simulink model "TosAeb"  (R2021a)
settings: Solver=FixedStepDiscrete  StartTime=0.0  StopTime=inf  FixedStep=0.01  RelTol=1e-3
undefined symbols (not defined in the file or in its folder): ACCModSts, AccSts1, AEB_ObjectMovingOrientation, AEB_ObjectMovingState, CN_BTCActive, CN_CurrentGearR, CN_CurrentGearR_DHT, CN_PTCActive, CN_VDCActive, CN_VSC_EspOff, CN_VSC_FCW_AEB_Error, CN_VSC_FCW_AEB_NoDispay, CN_VSC_FCW_AEB_PerfmDgdtn, CN_VSC_FCW_AEB_TempUnAvlbl, CN_VSC_VehStandstill, CNaccelAvoidMax, CNaccelAvoidMin, CNbrakePedalPress, CNcomfortStrLeftAccelMax, CNcomfortStrLeftAccelMin, CNcomfortStrRightAccelMax, CNcomfortStrRightAccelMin, CNDrvDoorOpen, CNDrvModeEco, CNDrvModeNorm, CNDrvModeOther, CNDrvModeSport, CNDrvSBRreminder, CNNull, CNobjInvalidID, CNPassDoorOpen, CNPassSBRreminder, CNPEBSAccelNegInf, CNPEBSAccelNull, CNPEBSTimeInf, CNPEBSTimeNull, CNSTOPMAX, CNSTOPNULL, CNsubjWidth, CNSwtOFF, CNSwtON, CNTTCMAX, CNTTCNULL, CNVehConfigAEBCycNone, CNVehConfigAEBNone, CNVehConfigAEBPedNone, CNVehConfigFCWNone, CNVehConfigJANone, CollisionSeverity, FALSE, FCWSnvty, JACycObjCalcInfoDefaultParams, JAPedObjCalcInfoDefaultParams, JAVehObjCalcInfoDefaultParams, latCycObjCalcInfoDefaultParams, latPedObjCalcInfoDefaultParams, longObjCalcInfoDefaultParams, ModSts, movingStatus, ObjectType ... (+1112)
libraries: simulink x3031, AEBSFunctionLib x50, Ta_Library x33, AEBSStateMachineLib x23, Mylibrary x12, simulink_extras x7, ObjSelect_lib x3, dspstat3 x2
(part 21 of 102)

== system TosAeb/AEB_Objsel/TA_Processor/PreProcessor/VehiclePreProcessor/CalculateCurvature/StaticBicycleModelC0/CalculateCurvatureCoG ==
  note: V^2/R  = w^2 R
      | M w^2 R/Cr = F/ Cr =
  note: We use a bicycle model with the following relations:
      |
      | Lf = Distance from CoG to front axle
      | Lr = Distance from CoG to rear axle
      | B = WheelBase = Lf + Lr
      | R = Curvature radius for CoG
      | M = Vehicle mass
      | V = Vehicle Speed
      | Cf = Cornering stiffness front
      | Cr = Cornering stiffness rear
      |
      | WheelAngle = RearSlipAngle - FrontSlipAngle + B/R
      | RearSlipAngle = - M Lf V^2 / ( B Cr R)
      | FrontSlipAngle = - M Lr V^2 / ( B Cf R )
      |
      | We solve for the curvature radius R, and use that Curvature = 1/R. Then
      | Curvature = WheelAngle B /(B^2 + M V^2 (Lr/Cf - Lf/Cr) )
  WheelAngle : Inport
  DistCoGToRearAxle : Inport  Port=2
  DistCoGToFrontAxle : Inport  Port=3
  MassSpeedSquaredDivWheelBase : Inport  Port=4
  Constant : Constant  Value=P_TA_PrmForVehWhlBase
  Constant1 : Constant  Value=single(1)  OutDataTypeStr=single
  Constant2 : Constant  Value=P_TA_PrmForVehBicycleMdlCornrgStfnFrnt
  Constant3 : Constant  Value=P_TA_PrmForVehBicycleMdlCornrgStfnRe
  Divide2 : Product  Tag=struct('comment',struct('text','','placement',1),'logdata',struct('maxnumvals',inf,'plotchannels',[],'loggingmode',3,'channels',1),'output',struct('variable','','description','','name','$S_$B','width',1,'address','','max',NaN,'min',NaN,'class','default','type','Float32','scaling','','lsb',1,'offset',0,'arb',0,'freeze',1,'unit','','autoscalingmode',1,'lowerheadroom',1,'upperheadroom',1,'headroomunit','bit','keepdatatype',1,'checkmin',0,'checkmax',0,'castoutput',0,'show',0),'inputs','**/','exceptionhandling',1,'scalingvalid',0,'date',734662.46776173613)  Inputs=**
  RadiusCoGJudge : Subsystem
  SafeDivider : Mylibrary/SafeDivider  SystemSampleTime=-1  VariantActivationTime=update diagram  Latency=0  AutoFrameSizeCalculation=off
  SafeDivider1 : Mylibrary/SafeDivider  SystemSampleTime=-1  VariantActivationTime=update diagram  Latency=0  AutoFrameSizeCalculation=off
  SafeDivider2 : Mylibrary/SafeDivider  SystemSampleTime=-1  VariantActivationTime=update diagram  Latency=0  AutoFrameSizeCalculation=off
  Sum1 : Sum  Tag=struct('comment',struct('text','','placement',1),'logdata',struct('maxnumvals',inf,'plotchannels',[],'loggingmode',3,'channels',1),'output',struct('variable','','description','','name','$S_$B','width',1,'address','','max',NaN,'min',NaN,'class','default','type','Float32','scaling','','lsb',1,'offset',0,'arb',0,'freeze',1,'unit','','autoscalingmode',1,'lowerheadroom',1,'upperheadroom',1,'headroomunit','bit','keepdatatype',1,'checkmin',0,'checkmax',0,'castoutput',0,'show',0),'inputs','++','scalingvalid',0,'date',730486)  InputSameDT=on  SaturateOnIntegerOverflow=on  OutDataTypeStr=Inherit: Same as first input
      settings: AttributesFormatString=InputSameDT=%<InputSameDT>
  Sum2 : Sum  Tag=struct('comment',struct('text','','placement',1),'logdata',struct('maxnumvals',inf,'plotchannels',[],'loggingmode',3,'channels',1),'output',struct('variable','','description','','name','$S_$B','width',1,'address','','max',NaN,'min',NaN,'class','default','type','Float32','scaling','','lsb',1,'offset',0,'arb',0,'freeze',1,'unit','','autoscalingmode',1,'lowerheadroom',1,'upperheadroom',1,'headroomunit','bit','keepdatatype',1,'checkmin',0,'checkmax',0,'castoutput',0,'show',0),'inputs','+-','scalingvalid',0,'date',734662.46684667829)  Inputs=+-  InputSameDT=on  SaturateOnIntegerOverflow=on  OutDataTypeStr=Inherit: Same as first input
      settings: AttributesFormatString=InputSameDT=%<InputSameDT>
  RadiusCoG : Outport
  CurvatureCoG : Outport  Port=2
  wires:
  SafeDivider1/1 -> Sum2/2
  DistCoGToFrontAxle/1 -> SafeDivider1/1
  WheelAngle/1 -> SafeDivider2/1
  SafeDivider/1 -> Sum2/1
  DistCoGToRearAxle/1 -> SafeDivider/1
  SafeDivider2/1 -> RadiusCoGJudge/2(CurvatureCoG), CurvatureCoG/1  "CurvatureCoG"
  Sum2/1 -> Divide2/1
  MassSpeedSquaredDivWheelBase/1 -> Divide2/2
  Divide2/1 -> Sum1/2
  Sum1/1 -> SafeDivider2/2
  Constant/1 -> Sum1/1
  Constant2/1 -> SafeDivider/2
  Constant3/1 -> SafeDivider1/2
  Constant1/1 -> RadiusCoGJudge/1(Con)
  RadiusCoGJudge/1(RadiusCoG) -> RadiusCoG/1

== system TosAeb/AEB_Objsel/TA_Processor/PreProcessor/VehiclePreProcessor/CalculateCurvature/StaticBicycleModelC0/CalculateCurvatureRear ==
  RadiusCOG : Inport
  DistCoGToRearAxle : Inport  Port=2
  SlipAngle : Inport  Port=3
  Constant : Constant  Value=single(1)  OutDataTypeStr=single
  Cos : Trigonometry  Operator=cos
  Multipl{,1..4} (5 alike) : Product  Tag=struct('comment',struct('text','','placement',1),'logdata',struct('maxnumvals',inf,'plotchannels',[],'loggingmode',3,'channels',1),'output',struct('variable','','description','','name','$S_$B','width',1,'address','','max',NaN,'min',NaN,'class','LOCAL','type','Float32','scaling','','lsb',1,'offset',0,'arb',0,'freeze',1,'unit','','autoscalingmode',1,'lowerheadroom',1,'upperheadroom',1,'headroomunit','bit','keepdatatype',1,'checkmin',0,'checkmax',0,'castoutput',0,'show',0),'inputs','****','exceptionhandling',1,'scalingvalid',0,'date',734725.68365415512)  InputSameDT=on  RndMeth=Zero  SaturateOnIntegerOverflow=on  OutDataTypeStr=Inherit: Same as first input
  SafeDivider : Mylibrary/SafeDivider  SystemSampleTime=-1  VariantActivationTime=update diagram  Latency=0  AutoFrameSizeCalculation=off
  Sign : Signum
  Sin : Trigonometry
  Sqrt : Sqrt  SaturateOnIntegerOverflow=on
  Sum1 : Sum  Tag=struct('comment',struct('text','','placement',1),'logdata',struct('maxnumvals',inf,'plotchannels',[],'loggingmode',3,'channels',1),'output',struct('variable','','description','','name','$S_$B','width',1,'address','','max',NaN,'min',NaN,'class','default','type','Float32','scaling','','lsb',1,'offset',0,'arb',0,'freeze',1,'unit','','autoscalingmode',1,'lowerheadroom',1,'upperheadroom',1,'headroomunit','bit','keepdatatype',1,'checkmin',0,'checkmax',0,'castoutput',0,'show',0),'inputs','+-','scalingvalid',0,'date',734662.46684667829)  Inputs=+-  InputSameDT=on  SaturateOnIntegerOverflow=on  OutDataTypeStr=Inherit: Same as first input
      settings: AttributesFormatString=InputSameDT=%<InputSameDT>
  Sum3 : Sum  Tag=struct('comment',struct('text','','placement',1),'logdata',struct('maxnumvals',inf,'plotchannels',[],'loggingmode',3,'channels',1),'output',struct('variable','','description','','name','$S_$B','width',1,'address','','max',NaN,'min',NaN,'class','default','type','Float32','scaling','','lsb',1,'offset',0,'arb',0,'freeze',1,'unit','','autoscalingmode',1,'lowerheadroom',1,'upperheadroom',1,'headroomunit','bit','keepdatatype',1,'checkmin',0,'checkmax',0,'castoutput',0,'show',0),'inputs','+-','scalingvalid',0,'date',734662.46684667829)  InputSameDT=on  SaturateOnIntegerOverflow=on  OutDataTypeStr=Inherit: Same as first input
      settings: AttributesFormatString=InputSameDT=%<InputSameDT>
  CurvatureRear : Outport
  wires:
  SlipAngle/1 -> Sin/1, Cos/1
  Sin/1 -> Multipl2/1
  Cos/1 -> Multipl3/1
  RadiusCOG/1 -> Multipl3/2, Sign/1, [RadiusCoG]
  Multipl3/1 -> Multipl/1, Multipl/2  "RadiusCentOfRot"
  DistCoGToRearAxle/1 -> Sum1/1
  Multipl2/1 -> Sum1/2
  Sum1/1 -> Multipl1/1, Multipl1/2
  Multipl1/1 -> Sum3/1
  Multipl/1 -> Sum3/2
  Sum3/1 -> Sqrt/1
  SafeDivider/1 -> CurvatureRear/1  "CurvatureRear"
  Sign/1 -> Multipl4/2
  Sqrt/1 -> Multipl4/1
  [RadiusCoG] -> Multipl2/2
  Multipl4/1 -> SafeDivider/2  "RadiusRear"
  Constant/1 -> SafeDivider/1

== system TosAeb/AEB_Objsel/TA_Processor/PreProcessor/VehiclePreProcessor/CalculateCurvature/StaticBicycleModelC0/CalculateDistanceToAxles ==
  note: 计算质心至后轴中心的距离
  Constant : Constant  Value=P_TA_PrmForVehWhlBase
  Constant1 : Constant  Value=P_TA_PrmForVehBicycleMdlAxleDistFrnt
  Rename : Subsystem  mask(Rename){}
      mask help: Block intended for fooling the model advisor, which want the signal names to match the port names and which only allows signal renaming in combination with transformative operations. The block has no effect on the signal and is intended to replace, e.g., gain blocks with one as its multiplication factor.
      mask Help: Block intended for fooling the model advisor, which want the signal names to match the port names and which only allows signal renaming in combination with transformative operations. The block has no effect on the signal and is intended to replace, e.g., gain blocks with one as its multiplication factor.
      mask Display:
      | % Author: Jakob Andersson (jande573)
      | 
      | % Display the block title
      | disp('Rename');
  Sum2 : Sum  Tag=struct('comment',struct('text','','placement',1),'logdata',struct('maxnumvals',inf,'plotchannels',[],'loggingmode',3,'channels',1),'output',struct('variable','','description','','name','$S_$B','width',1,'address','','max',NaN,'min',NaN,'class','default','type','Float32','scaling','','lsb',1,'offset',0,'arb',0,'freeze',1,'unit','','autoscalingmode',1,'lowerheadroom',1,'upperheadroom',1,'headroomunit','bit','keepdatatype',1,'checkmin',0,'checkmax',0,'castoutput',0,'show',0),'inputs','+-','scalingvalid',0,'date',734662.46684667829)  Inputs=+-  InputSameDT=on  SaturateOnIntegerOverflow=on  OutDataTypeStr=Inherit: Same as first input
      settings: AttributesFormatString=InputSameDT=%<InputSameDT>
  DistCoGToRearAxle : Outport
  DistCoGToFrontAxle : Outport  Port=2
  wires:
  Sum2/1 -> DistCoGToRearAxle/1  "DistCoGToRearAxle"
  Rename/1(Name_out) -> DistCoGToFrontAxle/1  "DistCoGToFrontAxle"
  Constant/1 -> Sum2/1
  Constant1/1 -> Rename/1(Name_in), Sum2/2

== system TosAeb/AEB_Objsel/TA_Processor/PreProcessor/VehiclePreProcessor/CalculateCurvature/StaticBicycleModelC0/CalculateSlip ==
  note: b/r表示后轴对应的角度值
      | m*v^2/（r*a) 表示后轮对应的角度值
      | 二者相减可得角贝塔
  CurvatureCoG : Inport
  DistCoGToRearAxle : Inport  Port=2
  DistCoGToFrontAxle : Inport  Port=3
  MassSpeedSquaredDivWheelBase : Inport  Port=4
  Constant3 : Constant  Value=P_TA_PrmForVehBicycleMdlCornrgStfnRe
  Multiply_1 : Product  Tag=struct('comment',struct('text','','placement',1),'logdata',struct('maxnumvals',inf,'plotchannels',[],'loggingmode',3,'channels',1),'output',struct('variable','','description','','name','$S_$B','width',1,'address','','max',NaN,'min',NaN,'class','LOCAL','type','Float32','scaling','','lsb',1,'offset',0,'arb',0,'freeze',1,'unit','','autoscalingmode',1,'lowerheadroom',1,'upperheadroom',1,'headroomunit','bit','keepdatatype',1,'checkmin',0,'checkmax',0,'castoutput',0,'show',0),'inputs','****','exceptionhandling',1,'scalingvalid',0,'date',734725.68365415512)  InputSameDT=on  RndMeth=Zero  SaturateOnIntegerOverflow=on  OutDataTypeStr=Inherit: Same as first input
  Multiply_3 : Product  Tag=struct('comment',struct('text','','placement',1),'logdata',struct('maxnumvals',inf,'plotchannels',[],'loggingmode',3,'channels',1),'output',struct('variable','','description','','name','$S_$B','width',1,'address','','max',NaN,'min',NaN,'class','LOCAL','type','Float32','scaling','','lsb',1,'offset',0,'arb',0,'freeze',1,'unit','','autoscalingmode',1,'lowerheadroom',1,'upperheadroom',1,'headroomunit','bit','keepdatatype',1,'checkmin',0,'checkmax',0,'castoutput',0,'show',0),'inputs','****','exceptionhandling',1,'scalingvalid',0,'date',734725.68365415512)  InputSameDT=on  RndMeth=Zero  SaturateOnIntegerOverflow=on  OutDataTypeStr=Inherit: Same as first input
  SafeDivider : Mylibrary/SafeDivider  SystemSampleTime=-1  VariantActivationTime=update diagram  Latency=0  AutoFrameSizeCalculation=off
  Sum3 : Sum  Tag=struct('comment',struct('text','','placement',1),'logdata',struct('maxnumvals',inf,'plotchannels',[],'loggingmode',3,'channels',1),'output',struct('variable','','description','','name','$S_$B','width',1,'address','','max',NaN,'min',NaN,'class','default','type','Float32','scaling','','lsb',1,'offset',0,'arb',0,'freeze',1,'unit','','autoscalingmode',1,'lowerheadroom',1,'upperheadroom',1,'headroomunit','bit','keepdatatype',1,'checkmin',0,'checkmax',0,'castoutput',0,'show',0),'inputs','+-','scalingvalid',0,'date',734662.46684667829)  Inputs=+-  InputSameDT=on  SaturateOnIntegerOverflow=on  OutDataTypeStr=Inherit: Same as first input
      settings: AttributesFormatString=InputSameDT=%<InputSameDT>
  SlipAngle : Outport
  wires:
  CurvatureCoG/1 -> Multiply_3/1
  DistCoGToRearAxle/1 -> Sum3/1
  Multiply_1/1 -> Sum3/2
  MassSpeedSquaredDivWheelBase/1 -> Multiply_1/1
  Sum3/1 -> Multiply_3/2
  DistCoGToFrontAxle/1 -> SafeDivider/1
  Multiply_3/1 -> SlipAngle/1  "SlipAngle"
  SafeDivider/1 -> Multiply_1/2
  Constant3/1 -> SafeDivider/2

== system TosAeb/AEB_Objsel/TA_Processor/PreProcessor/VehiclePreProcessor/CalculateCurvature/StaticBicycleModelC0/CalculateWheelAngle ==
  PinionSteerAg : Inport
  Constant : Constant  Value=P_TA_PrmForVehSteerWhlAgRat
  SafeDivide : Subsystem  TreatAsAtomicUnit=on  mask(Safe divide){DataType=single, DataTypeMax=0, DataTypeMin=0}
      mask prompts: DataType "Data type:"
      mask help:
      | This block offers division protected from division by zero.
      |
      | When division by zero is detected, a very large number will be returned with the same sign as the numerator.
      |
      | In the case zero divided by zero, the output has been defined as zero.
      |
      | Both floating-point numbers and integers are supported.
      mask init: same as TosAeb/AEB_Objsel/TA_Processor/PreProcessor/VehiclePreProcessor/CalculateCurvature/SafeDivide
      settings: RTWSystemCode=Inline
      mask Help:
      | This block offers division protected from division by zero.
      | 
      | When division by zero is detected, a very large number will be returned with the same sign as the numerator.
      | 
      | In the case zero divided by zero, the output has been defined as zero.
      | 
      | Both floating-point numbers and integers are supported.
      mask Display:
      | % Author: Jakob Andersson (jande573)
      | 
      | % Display the allowed data type
      | disp(DataType);
      | 
      | % Display the port symbols
      | port_label('input',1,'\bullet','texmode','on');
      | port_label('input',2,'\div','texmode','on');
  WheelAngle : Outport
  wires:
  PinionSteerAg/1 -> SafeDivide/1(Numerator)
  SafeDivide/1(Quotient) -> WheelAngle/1  "WheelAngle"
  Constant/1 -> SafeDivide/2(Denominator)

== system TosAeb/AEB_Objsel/TA_Processor/PreProcessor/VehiclePreProcessor/CalculateCurvature/StaticBicycleModelC0/IntermediateCalculation ==
  note: 整车质量
      | 整车轴距
  note: 质量乘以速度的平方除以轴距
  note: m*v^2/Base
  Speed : Inport
  Constant : Constant  Value=P_TA_PrmForVehWholeWeight
  Constant1 : Constant  Value=P_TA_PrmForVehWhlBase
  Multiply_1 : Product  Tag=struct('comment',struct('text','','placement',1),'logdata',struct('maxnumvals',inf,'plotchannels',[],'loggingmode',3,'channels',1),'output',struct('variable','','description','','name','$S_$B','width',1,'address','','max',NaN,'min',NaN,'class','LOCAL','type','Float32','scaling','','lsb',1,'offset',0,'arb',0,'freeze',1,'unit','','autoscalingmode',1,'lowerheadroom',1,'upperheadroom',1,'headroomunit','bit','keepdatatype',1,'checkmin',0,'checkmax',0,'castoutput',0,'show',0),'inputs','****','exceptionhandling',1,'scalingvalid',0,'date',734725.68365415512)  Inputs=***  InputSameDT=on  RndMeth=Zero  SaturateOnIntegerOverflow=on  OutDataTypeStr=Inherit: Same as first input
  SafeDivide : Subsystem  TreatAsAtomicUnit=on  mask(Safe divide){DataType=single, DataTypeMax=0, DataTypeMin=0}
      mask prompts: DataType "Data type:"
      mask help:
      | This block offers division protected from division by zero.
      |
      | When division by zero is detected, a very large number will be returned with the same sign as the numerator.
      |
      | In the case zero divided by zero, the output has been defined as zero.
      |
      | Both floating-point numbers and integers are supported.
      mask init: same as TosAeb/AEB_Objsel/TA_Processor/PreProcessor/VehiclePreProcessor/CalculateCurvature/SafeDivide
      settings: RTWSystemCode=Inline
      mask Help:
      | This block offers division protected from division by zero.
      | 
      | When division by zero is detected, a very large number will be returned with the same sign as the numerator.
      | 
      | In the case zero divided by zero, the output has been defined as zero.
      | 
      | Both floating-point numbers and integers are supported.
      mask Display:
      | % Author: Jakob Andersson (jande573)
      | 
      | % Display the allowed data type
      | disp(DataType);
      | 
      | % Display the port symbols
      | port_label('input',1,'\bullet','texmode','on');
      | port_label('input',2,'\div','texmode','on');
  MassSpeedSquaredDivWheelBase : Outport
  wires:
  SafeDivide/1(Quotient) -> MassSpeedSquaredDivWheelBase/1  "MassSpeedSquaredDivWheelBase"
  Multiply_1/1 -> SafeDivide/1(Numerator)
  Speed/1 -> Multiply_1/1, Multiply_1/2
  Constant/1 -> Multiply_1/3
  Constant1/1 -> SafeDivide/2(Denominator)

== system TosAeb/AEB_Objsel/TA_Processor/PreProcessor/VehiclePreProcessor/CalculateCurvature/StaticBicycleModelC1/CalculateCurvatureCoG ==
  note: V^2/R  = w^2 R
      | M w^2 R/Cr = F/ Cr =
  note: We use a bicycle model with the following relations:
      |
      | Lf = Distance from CoG to front axle
      | Lr = Distance from CoG to rear axle
      | B = WheelBase = Lf + Lr
      | R = Curvature radius for CoG
      | M = Vehicle mass
      | V = Vehicle Speed
      | Cf = Cornering stiffness front
      | Cr = Cornering stiffness rear
      |
      | WheelAngle = RearSlipAngle - FrontSlipAngle + B/R
      | RearSlipAngle = - M Lf V^2 / ( B Cr R)
      | FrontSlipAngle = - M Lr V^2 / ( B Cf R )
      |
      | We solve for the curvature radius R, and use that Curvature = 1/R. Then
      | Curvature = WheelAngle B /(B^2 + M V^2 (Lr/Cf - Lf/Cr) )
  WheelAngle : Inport
  DistCoGToRearAxle : Inport  Port=2
  DistCoGToFrontAxle : Inport  Port=3
  MassSpeedSquaredDivWheelBase : Inport  Port=4
  Constant : Constant  Value=P_TA_PrmForVehWhlBase
  Constant1 : Constant  Value=single(1)  OutDataTypeStr=single
  Constant2 : Constant  Value=P_TA_PrmForVehBicycleMdlCornrgStfnFrnt
  Constant3 : Constant  Value=P_TA_PrmForVehBicycleMdlCornrgStfnRe
  Divide2 : Product  Tag=struct('comment',struct('text','','placement',1),'logdata',struct('maxnumvals',inf,'plotchannels',[],'loggingmode',3,'channels',1),'output',struct('variable','','description','','name','$S_$B','width',1,'address','','max',NaN,'min',NaN,'class','default','type','Float32','scaling','','lsb',1,'offset',0,'arb',0,'freeze',1,'unit','','autoscalingmode',1,'lowerheadroom',1,'upperheadroom',1,'headroomunit','bit','keepdatatype',1,'checkmin',0,'checkmax',0,'castoutput',0,'show',0),'inputs','**/','exceptionhandling',1,'scalingvalid',0,'date',734662.46776173613)  Inputs=**
  SafeDivider{,1..3} (4 alike) : Mylibrary/SafeDivider  SystemSampleTime=-1  VariantActivationTime=update diagram  Latency=0  AutoFrameSizeCalculation=off
  Sum1 : Sum  Tag=struct('comment',struct('text','','placement',1),'logdata',struct('maxnumvals',inf,'plotchannels',[],'loggingmode',3,'channels',1),'output',struct('variable','','description','','name','$S_$B','width',1,'address','','max',NaN,'min',NaN,'class','default','type','Float32','scaling','','lsb',1,'offset',0,'arb',0,'freeze',1,'unit','','autoscalingmode',1,'lowerheadroom',1,'upperheadroom',1,'headroomunit','bit','keepdatatype',1,'checkmin',0,'checkmax',0,'castoutput',0,'show',0),'inputs','++','scalingvalid',0,'date',730486)  InputSameDT=on  SaturateOnIntegerOverflow=on  OutDataTypeStr=Inherit: Same as first input
      settings: AttributesFormatString=InputSameDT=%<InputSameDT>
  Sum2 : Sum  Tag=struct('comment',struct('text','','placement',1),'logdata',struct('maxnumvals',inf,'plotchannels',[],'loggingmode',3,'channels',1),'output',struct('variable','','description','','name','$S_$B','width',1,'address','','max',NaN,'min',NaN,'class','default','type','Float32','scaling','','lsb',1,'offset',0,'arb',0,'freeze',1,'unit','','autoscalingmode',1,'lowerheadroom',1,'upperheadroom',1,'headroomunit','bit','keepdatatype',1,'checkmin',0,'checkmax',0,'castoutput',0,'show',0),'inputs','+-','scalingvalid',0,'date',734662.46684667829)  Inputs=+-  InputSameDT=on  SaturateOnIntegerOverflow=on  OutDataTypeStr=Inherit: Same as first input
      settings: AttributesFormatString=InputSameDT=%<InputSameDT>
  RadiusCoG : Outport
  CurvatureCoG : Outport  Port=2
  wires:
  Constant1/1 -> SafeDivider3/1
  SafeDivider1/1 -> Sum2/2
  SafeDivider3/1 -> RadiusCoG/1  "RadiusCoG"
  DistCoGToFrontAxle/1 -> SafeDivider1/1
  WheelAngle/1 -> SafeDivider2/1
  SafeDivider/1 -> Sum2/1
  DistCoGToRearAxle/1 -> SafeDivider/1
  SafeDivider2/1 -> SafeDivider3/2, CurvatureCoG/1  "CurvatureCoG"
  Sum2/1 -> Divide2/1
  MassSpeedSquaredDivWheelBase/1 -> Divide2/2
  Divide2/1 -> Sum1/2
  Sum1/1 -> SafeDivider2/2
  Constant/1 -> Sum1/1
  Constant2/1 -> SafeDivider/2
  Constant3/1 -> SafeDivider1/2

== system TosAeb/AEB_Objsel/TA_Processor/PreProcessor/VehiclePreProcessor/CalculateCurvature/StaticBicycleModelC1/CalculateCurvatureRear ==
  RadiusCOG : Inport
  DistCoGToRearAxle : Inport  Port=2
  SlipAngle : Inport  Port=3
  Constant : Constant  Value=single(1)  OutDataTypeStr=single
  Cos : Trigonometry  Operator=cos
  Multipl{,1..4} (5 alike) : Product  Tag=struct('comment',struct('text','','placement',1),'logdata',struct('maxnumvals',inf,'plotchannels',[],'loggingmode',3,'channels',1),'output',struct('variable','','description','','name','$S_$B','width',1,'address','','max',NaN,'min',NaN,'class','LOCAL','type','Float32','scaling','','lsb',1,'offset',0,'arb',0,'freeze',1,'unit','','autoscalingmode',1,'lowerheadroom',1,'upperheadroom',1,'headroomunit','bit','keepdatatype',1,'checkmin',0,'checkmax',0,'castoutput',0,'show',0),'inputs','****','exceptionhandling',1,'scalingvalid',0,'date',734725.68365415512)  InputSameDT=on  RndMeth=Zero  SaturateOnIntegerOverflow=on  OutDataTypeStr=Inherit: Same as first input
  SafeDivider1 : Mylibrary/SafeDivider  SystemSampleTime=-1  VariantActivationTime=update diagram  Latency=0  AutoFrameSizeCalculation=off
  Sign : Signum
  Sin : Trigonometry
  Sqrt : Sqrt  SaturateOnIntegerOverflow=on
  Sum1 : Sum  Tag=struct('comment',struct('text','','placement',1),'logdata',struct('maxnumvals',inf,'plotchannels',[],'loggingmode',3,'channels',1),'output',struct('variable','','description','','name','$S_$B','width',1,'address','','max',NaN,'min',NaN,'class','default','type','Float32','scaling','','lsb',1,'offset',0,'arb',0,'freeze',1,'unit','','autoscalingmode',1,'lowerheadroom',1,'upperheadroom',1,'headroomunit','bit','keepdatatype',1,'checkmin',0,'checkmax',0,'castoutput',0,'show',0),'inputs','+-','scalingvalid',0,'date',734662.46684667829)  Inputs=+-  InputSameDT=on  SaturateOnIntegerOverflow=on  OutDataTypeStr=Inherit: Same as first input
      settings: AttributesFormatString=InputSameDT=%<InputSameDT>
  Sum3 : Sum  Tag=struct('comment',struct('text','','placement',1),'logdata',struct('maxnumvals',inf,'plotchannels',[],'loggingmode',3,'channels',1),'output',struct('variable','','description','','name','$S_$B','width',1,'address','','max',NaN,'min',NaN,'class','default','type','Float32','scaling','','lsb',1,'offset',0,'arb',0,'freeze',1,'unit','','autoscalingmode',1,'lowerheadroom',1,'upperheadroom',1,'headroomunit','bit','keepdatatype',1,'checkmin',0,'checkmax',0,'castoutput',0,'show',0),'inputs','+-','scalingvalid',0,'date',734662.46684667829)  InputSameDT=on  SaturateOnIntegerOverflow=on  OutDataTypeStr=Inherit: Same as first input
      settings: AttributesFormatString=InputSameDT=%<InputSameDT>
  CurvatureRear : Outport
  wires:
  SlipAngle/1 -> Sin/1, Cos/1
  Sin/1 -> Multipl2/1
  Cos/1 -> Multipl3/1
  RadiusCOG/1 -> Multipl3/2, Sign/1, [RadiusCoG]
  Multipl3/1 -> Multipl/1, Multipl/2  "RadiusCentOfRot"
  DistCoGToRearAxle/1 -> Sum1/1
  Multipl2/1 -> Sum1/2
  Sum1/1 -> Multipl1/1, Multipl1/2
  Multipl1/1 -> Sum3/1
  Multipl/1 -> Sum3/2
  Sum3/1 -> Sqrt/1
  SafeDivider1/1 -> CurvatureRear/1  "CurvatureRear"
  Sign/1 -> Multipl4/2
  Sqrt/1 -> Multipl4/1
  [RadiusCoG] -> Multipl2/2
  Multipl4/1 -> SafeDivider1/2  "RadiusRear"
  Constant/1 -> SafeDivider1/1

== system TosAeb/AEB_Objsel/TA_Processor/PreProcessor/VehiclePreProcessor/CalculateCurvature/StaticBicycleModelC1/CalculateDistanceToAxles ==
  note: 计算质心至后轴中心的距离
  Constant : Constant  Value=P_TA_PrmForVehWhlBase
  Constant1 : Constant  Value=P_TA_PrmForVehBicycleMdlAxleDistFrnt
  Rename : Subsystem  mask(Rename){}
      mask help: Block intended for fooling the model advisor, which want the signal names to match the port names and which only allows signal renaming in combination with transformative operations. The block has no effect on the signal and is intended to replace, e.g., gain blocks with one as its multiplication factor.
      mask Help: Block intended for fooling the model advisor, which want the signal names to match the port names and which only allows signal renaming in combination with transformative operations. The block has no effect on the signal and is intended to replace, e.g., gain blocks with one as its multiplication factor.
      mask Display:
      | % Author: Jakob Andersson (jande573)
      | 
      | % Display the block title
      | disp('Rename');
  Sum2 : Sum  Tag=struct('comment',struct('text','','placement',1),'logdata',struct('maxnumvals',inf,'plotchannels',[],'loggingmode',3,'channels',1),'output',struct('variable','','description','','name','$S_$B','width',1,'address','','max',NaN,'min',NaN,'class','default','type','Float32','scaling','','lsb',1,'offset',0,'arb',0,'freeze',1,'unit','','autoscalingmode',1,'lowerheadroom',1,'upperheadroom',1,'headroomunit','bit','keepdatatype',1,'checkmin',0,'checkmax',0,'castoutput',0,'show',0),'inputs','+-','scalingvalid',0,'date',734662.46684667829)  Inputs=+-  InputSameDT=on  SaturateOnIntegerOverflow=on  OutDataTypeStr=Inherit: Same as first input
      settings: AttributesFormatString=InputSameDT=%<InputSameDT>
  DistCoGToRearAxle : Outport
  DistCoGToFrontAxle : Outport  Port=2
  wires:
  Sum2/1 -> DistCoGToRearAxle/1  "DistCoGToRearAxle"
  Rename/1(Name_out) -> DistCoGToFrontAxle/1  "DistCoGToFrontAxle"
  Constant/1 -> Sum2/1
  Constant1/1 -> Rename/1(Name_in), Sum2/2

== system TosAeb/AEB_Objsel/TA_Processor/PreProcessor/VehiclePreProcessor/CalculateCurvature/StaticBicycleModelC1/CalculateSlip ==
  CurvatureCoG : Inport
  DistCoGToRearAxle : Inport  Port=2
  DistCoGToFrontAxle : Inport  Port=3
  MassSpeedSquaredDivWheelBase : Inport  Port=4
  Constant3 : Constant  Value=P_TA_PrmForVehBicycleMdlCornrgStfnRe
  Multiply_1 : Product  Tag=struct('comment',struct('text','','placement',1),'logdata',struct('maxnumvals',inf,'plotchannels',[],'loggingmode',3,'channels',1),'output',struct('variable','','description','','name','$S_$B','width',1,'address','','max',NaN,'min',NaN,'class','LOCAL','type','Float32','scaling','','lsb',1,'offset',0,'arb',0,'freeze',1,'unit','','autoscalingmode',1,'lowerheadroom',1,'upperheadroom',1,'headroomunit','bit','keepdatatype',1,'checkmin',0,'checkmax',0,'castoutput',0,'show',0),'inputs','****','exceptionhandling',1,'scalingvalid',0,'date',734725.68365415512)  InputSameDT=on  RndMeth=Zero  SaturateOnIntegerOverflow=on  OutDataTypeStr=Inherit: Same as first input
  Multiply_3 : Product  Tag=struct('comment',struct('text','','placement',1),'logdata',struct('maxnumvals',inf,'plotchannels',[],'loggingmode',3,'channels',1),'output',struct('variable','','description','','name','$S_$B','width',1,'address','','max',NaN,'min',NaN,'class','LOCAL','type','Float32','scaling','','lsb',1,'offset',0,'arb',0,'freeze',1,'unit','','autoscalingmode',1,'lowerheadroom',1,'upperheadroom',1,'headroomunit','bit','keepdatatype',1,'checkmin',0,'checkmax',0,'castoutput',0,'show',0),'inputs','****','exceptionhandling',1,'scalingvalid',0,'date',734725.68365415512)  InputSameDT=on  RndMeth=Zero  SaturateOnIntegerOverflow=on  OutDataTypeStr=Inherit: Same as first input
  SafeDivider : Mylibrary/SafeDivider  SystemSampleTime=-1  VariantActivationTime=update diagram  Latency=0  AutoFrameSizeCalculation=off
  Sum3 : Sum  Tag=struct('comment',struct('text','','placement',1),'logdata',struct('maxnumvals',inf,'plotchannels',[],'loggingmode',3,'channels',1),'output',struct('variable','','description','','name','$S_$B','width',1,'address','','max',NaN,'min',NaN,'class','default','type','Float32','scaling','','lsb',1,'offset',0,'arb',0,'freeze',1,'unit','','autoscalingmode',1,'lowerheadroom',1,'upperheadroom',1,'headroomunit','bit','keepdatatype',1,'checkmin',0,'checkmax',0,'castoutput',0,'show',0),'inputs','+-','scalingvalid',0,'date',734662.46684667829)  Inputs=+-  InputSameDT=on  SaturateOnIntegerOverflow=on  OutDataTypeStr=Inherit: Same as first input
      settings: AttributesFormatString=InputSameDT=%<InputSameDT>
  SlipAngle : Outport
  wires:
  CurvatureCoG/1 -> Multiply_3/1
  DistCoGToRearAxle/1 -> Sum3/1
  Multiply_1/1 -> Sum3/2
  MassSpeedSquaredDivWheelBase/1 -> Multiply_1/1
  Sum3/1 -> Multiply_3/2
  DistCoGToFrontAxle/1 -> SafeDivider/1
  Multiply_3/1 -> SlipAngle/1  "SlipAngle"
  SafeDivider/1 -> Multiply_1/2
  Constant3/1 -> SafeDivider/2

== system TosAeb/AEB_Objsel/TA_Processor/PreProcessor/VehiclePreProcessor/CalculateCurvature/StaticBicycleModelC1/CalculateWheelAngle ==
  PinionSteerAgRate : Inport
  Constant : Constant  Value=P_TA_PrmForVehSteerWhlAgRat
  SafeDivide : Subsystem  TreatAsAtomicUnit=on  mask(Safe divide){DataType=single, DataTypeMax=0, DataTypeMin=0}
      mask prompts: DataType "Data type:"
      mask help:
      | This block offers division protected from division by zero.
      |
      | When division by zero is detected, a very large number will be returned with the same sign as the numerator.
      |
      | In the case zero divided by zero, the output has been defined as zero.
      |
      | Both floating-point numbers and integers are supported.
      mask init: same as TosAeb/AEB_Objsel/TA_Processor/PreProcessor/VehiclePreProcessor/CalculateCurvature/SafeDivide
      settings: RTWSystemCode=Inline
      mask Help:
      | This block offers division protected from division by zero.
      | 
      | When division by zero is detected, a very large number will be returned with the same sign as the numerator.
      | 
      | In the case zero divided by zero, the output has been defined as zero.
      | 
      | Both floating-point numbers and integers are supported.
      mask Display:
      | % Author: Jakob Andersson (jande573)
      | 
      | % Display the allowed data type
      | disp(DataType);
      | 
      | % Display the port symbols
      | port_label('input',1,'\bullet','texmode','on');
      | port_label('input',2,'\div','texmode','on');
  WheelAngle : Outport
  wires:
  PinionSteerAgRate/1 -> SafeDivide/1(Numerator)
  SafeDivide/1(Quotient) -> WheelAngle/1  "WheelAngle"
  Constant/1 -> SafeDivide/2(Denominator)

== system TosAeb/AEB_Objsel/TA_Processor/PreProcessor/VehiclePreProcessor/CalculateCurvature/StaticBicycleModelC1/IntermediateCalculation ==
  note: 整车质量
      | 整车轴距
  note: 质量乘以速度的平方除以轴距
  note: m*v^2/Base
  Speed : Inport
  Constant : Constant  Value=P_TA_PrmForVehWholeWeight
  Constant1 : Constant  Value=P_TA_PrmForVehWhlBase
  Multiply_1 : Product  Tag=struct('comment',struct('text','','placement',1),'logdata',struct('maxnumvals',inf,'plotchannels',[],'loggingmode',3,'channels',1),'output',struct('variable','','description','','name','$S_$B','width',1,'address','','max',NaN,'min',NaN,'class','LOCAL','type','Float32','scaling','','lsb',1,'offset',0,'arb',0,'freeze',1,'unit','','autoscalingmode',1,'lowerheadroom',1,'upperheadroom',1,'headroomunit','bit','keepdatatype',1,'checkmin',0,'checkmax',0,'castoutput',0,'show',0),'inputs','****','exceptionhandling',1,'scalingvalid',0,'date',734725.68365415512)  Inputs=***  InputSameDT=on  RndMeth=Zero  SaturateOnIntegerOverflow=on  OutDataTypeStr=Inherit: Same as first input
  SafeDivide : Subsystem  TreatAsAtomicUnit=on  mask(Safe divide){DataType=single, DataTypeMax=0, DataTypeMin=0}
      mask prompts: DataType "Data type:"
      mask help:
      | This block offers division protected from division by zero.
      |
      | When division by zero is detected, a very large number will be returned with the same sign as the numerator.
      |
      | In the case zero divided by zero, the output has been defined as zero.
      |
      | Both floating-point numbers and integers are supported.
      mask init: same as TosAeb/AEB_Objsel/TA_Processor/PreProcessor/VehiclePreProcessor/CalculateCurvature/SafeDivide
      settings: RTWSystemCode=Inline
      mask Help:
      | This block offers division protected from division by zero.
      | 
      | When division by zero is detected, a very large number will be returned with the same sign as the numerator.
      | 
      | In the case zero divided by zero, the output has been defined as zero.
      | 
      | Both floating-point numbers and integers are supported.
      mask Display:
      | % Author: Jakob Andersson (jande573)
      | 
      | % Display the allowed data type
      | disp(DataType);
      | 
      | % Display the port symbols
      | port_label('input',1,'\bullet','texmode','on');
      | port_label('input',2,'\div','texmode','on');
  MassSpeedSquaredDivWheelBase : Outport
  wires:
  SafeDivide/1(Quotient) -> MassSpeedSquaredDivWheelBase/1  "MassSpeedSquaredDivWheelBase"
  Multiply_1/1 -> SafeDivide/1(Numerator)
  Speed/1 -> Multiply_1/1, Multiply_1/2
  Constant/1 -> Multiply_1/3
  Constant1/1 -> SafeDivide/2(Denominator)

== system TosAeb/AEB_Objsel/TA_Processor/PreProcessor/VehiclePreProcessor/PathEstimn_Calculate/For Iterator PahEstimn/CheckVehicleStopping ==
  VehicleMotionT0 : Inport
  Abs : Abs
  Add : Sum  AccumDataTypeStr=single  OutDataTypeStr=single
  Add1 : Sum  OutDataTypeStr=single
  Bus Creator : BusCreator  Inputs=3
  Bus Selector : BusSelector  OutputSignals=Speed
  Bus Selector1 : BusSelector  OutputSignals=A
  Bus Selector2 : BusSelector  OutputSignals=VelocityLongitudinal
  Bus Selector3 : BusSelector  OutputSignals=AccelerationLongitudinal
  Bus Selector4 : BusSelector  OutputSignals=VelocityLateral
  Bus Selector5 : BusSelector  OutputSignals=AccelerationLateral
  Compare To Constant : simulink/Logic and Bit Operations/Compare To Constant  relop=<  const=1  OutDataTypeStr=boolean
  Compare To Zero : simulink/Logic and Bit Operations/Compare To Zero  relop=>  OutDataTypeStr=boolean
  Constant : Constant  Value=P_DM_VehSelfMinSpdInDrvrStEstimr
  Constant1 : Constant  Value=single(0.1)
  Constant2 : Constant  Value=single(0)
  Constant3 : Constant  Value=single(0)
  Gain : Gain  Gain=single(0.5)
  Logical Operator : Logic  AllPortsSameDT=off  OutDataTypeStr=boolean
  Product{,1..3} (4 alike) : Product
  Relational Operator : RelationalOperator  Operator=<=  OutDataTypeStr=boolean  RndMeth=Simplest
  SafetyDivide : Mylibrary/SafeDivide  DataType=single  DataTypeMax=0  DataTypeMin=0
  Saturation : Saturate  UpperLimit=4  LowerLimit=0
  Square : Math  Operator=square  SaturateOnIntegerOverflow=on
  Square1 : Math  Operator=square  SaturateOnIntegerOverflow=on
  Switch : Switch  Criteria=u2 ~= 0
  Switch1 : Switch  Criteria=u2 ~= 0
  Switch2 : Switch  Criteria=u2 ~= 0
  Unary Minus : UnaryMinus
  StoppingDistances : Outport
  wires:
  VehicleMotionT0/1 -> Bus Selector3/1, Bus Selector4/1, Bus Selector5/1, Bus Selector2/1, Bus Selector1/1, Bus Selector/1
  Bus Selector/1 -> SafetyDivide/1, Abs/1  "<Speed>"
  Abs/1 -> Relational Operator/1
  Constant/1 -> Relational Operator/2
  Relational Operator/1 -> [VehicleHasStopped]  "VehicleHasStopped"
  Bus Selector1/1 -> Unary Minus/1  "<A>"
  Unary Minus/1 -> SafetyDivide/2
  SafetyDivide/1 -> Saturation/1
  Saturation/1 -> [StoppingTime], Switch/3
  [VehicleHasStopped] -> Switch/2
  Constant1/1 -> Switch/1
  Switch/1 -> Compare To Constant/1, Compare To Zero/1
  Compare To Constant/1 -> Logical Operator/2
  Compare To Zero/1 -> Logical Operator/1
  Bus Selector2/1 -> Product/1  "<VelocityLongitudinal>"
  [StoppingTime] -> Product/2
  Bus Selector3/1 -> Gain/1  "<AccelerationLongitudinal>"
  Gain/1 -> Product1/1
  [StoppingTime] -> Square/1
  Square/1 -> Product1/2
  Product/1 -> Add/1
  Product1/1 -> Add/2
  Add/1 -> Switch1/3
  [VehicleHasStopped] -> Switch1/2
  Constant2/1 -> Switch1/1
  Bus Selector4/1 -> Product2/1  "<VelocityLateral>"
  [StoppingTime] -> Product2/2
  Bus Selector5/1 -> Product3/1  "<AccelerationLateral>"
  [StoppingTime] -> Square1/1
  Square1/1 -> Product3/2
  Product2/1 -> Add1/1
  Product3/1 -> Add1/2
  Add1/1 -> Switch2/3
  Constant3/1 -> Switch2/1
  [VehicleHasStopped] -> Switch2/2
  Logical Operator/1 -> Bus Creator/1  "StopsWithinSegment"
  Switch1/1 -> Bus Creator/2  "LongitudinalStoppingDistance"
  Switch2/1 -> Bus Creator/3  "LateralStoppingDistance"
  Bus Creator/1 -> StoppingDistances/1

== system TosAeb/AEB_Objsel/TA_Processor/PreProcessor/VehiclePreProcessor/PathEstimn_Calculate/For Iterator PahEstimn/ComputeVehicleStateAtT1 ==
  VehicleMotionT0 : Inport
  StoppingDistances : Inport  Port=2
  ControlPoints : Inport  Port=3
  AccelerationAtT1 : Subsystem
  Add1 : Sum
  Add2 : Sum
  Bus Creator : BusCreator  Inputs=10
  Bus Selector : BusSelector  OutputSignals=Speed,A
  Bus Selector1 : BusSelector  OutputSignals=StopsWithinSegment
  Bus Selector2 : BusSelector  OutputSignals=P1Longitudinal,P2Longitudinal,P3Longitudinal,P1Lateral,P2Lateral,P3Lateral
  Bus Selector3 : BusSelector  OutputSignals=HeadingAngle
  Bus Selector4 : BusSelector  OutputSignals=Curvature,CurvatureRate
  ComputeHeadingAngle : Subsystem
  MATLAB Function : MATLAB Function  TreatAsAtomicUnit=on
  Saturation1 : Saturate
  Saturation2 : Saturate  UpperLimit=inf  LowerLimit=P_DM_LoPosValInDrvrStEstimr
  c : Subsystem
  VehicleMotionT1 : Outport
  wires:
  Bus Selector/1 -> Add2/1  "<Speed>"
  Bus Selector/2 -> Add2/2, AccelerationAtT1/1(AT0)  "<A>"
  Add2/1 -> Saturation2/1  "Speed"
  StoppingDistances/1 -> Bus Selector1/1
  Bus Selector1/1 -> AccelerationAtT1/2(StopsWithinSegment)  "<StopsWithinSegment>"
  ControlPoints/1 -> Bus Selector2/1
  Bus Selector2/1 -> c/1(P1Longitudinal)  "<P1Longitudinal>"
  Bus Selector2/2 -> c/2(P2Longitudinal)  "<P2Longitudinal>"
  Bus Selector2/3 -> c/3(P3Longitudinal)  "<P3Longitudinal>"
  Bus Selector2/4 -> c/4(P1Lateral)  "<P1Lateral>"
  Bus Selector2/5 -> c/5(P2Lateral)  "<P2Lateral>"
  Bus Selector2/6 -> c/6(P3Lateral)  "<P3Lateral>"
  [VehicleMotionT0] -> Bus Selector3/1
  c/4(VLat) -> ComputeHeadingAngle/2(VLat)
  Bus Selector3/1 -> ComputeHeadingAngle/3(HeadingAngleT0)  "<HeadingAngle>"
  c/1(PosnLgt) -> [PosnLgt]
  [VehicleMotionT0] -> Bus Selector4/1
  Bus Selector4/1 -> Add1/1  "<Curvature>"
  Bus Selector4/2 -> Add1/2  "<CurvatureRate>"
  Add1/1 -> Saturation1/1  "Curvature"
  ComputeHeadingAngle/1(HeadingAngle) -> [HeadingAngle], MATLAB Function/3(HeadingAngle)
  AccelerationAtT1/1(A) -> Bus Creator/4, MATLAB Function/1(A)  "A"
  [Speed] -> MATLAB Function/2(Speed)
  Saturation1/1 -> MATLAB Function/4(Curvature), Bus Creator/9  "Curvature"
  MATLAB Function/4(ALat) -> Bus Creator/8  "AccelerationLateral"
  MATLAB Function/3(ALgt) -> Bus Creator/7  "AccelerationLongitudinal"
  MATLAB Function/2(VLat) -> Bus Creator/6  "VelocityLateral"
  MATLAB Function/1(VLgt) -> Bus Creator/5  "VelocityLongitudinal"
  [PosnLgt] -> Bus Creator/1  "PositionLongitudinal"
  c/3(VLgt) -> ComputeHeadingAngle/1(VLgt)
  c/2(PosnLat) -> [PosnLat]
  [PosnLat] -> Bus Creator/2  "PositionLateral"
  [HeadingAngle] -> Bus Creator/10  "HeadingAngle"
  Bus Creator/1 -> VehicleMotionT1/1  "VehicleMotionT1"
  VehicleMotionT0/1 -> Bus Selector/1, [VehicleMotionT0]
  Saturation2/1 -> Bus Creator/3, [Speed]  "Speed"

== system TosAeb/AEB_Objsel/TA_Processor/PreProcessor/VehiclePreProcessor/PathEstimn_Calculate/For Iterator PahEstimn/InitialControlPointsCalculation ==
  StoppingDistances : Inport
  VehicleMotionT0 : Inport  Port=2
  Bus Creator : BusCreator  Inputs=8
  Bus Selector : BusSelector  OutputSignals=PositionLongitudinal,VelocityLongitudinal,AccelerationLongitudinal,PositionLateral,VelocityLateral,AccelerationLateral
  Bus Selector1 : BusSelector  OutputSignals=PositionLongitudinal,PositionLateral,Speed,A,HeadingAngle,Curvature,CurvatureRate
  Bus Selector2 : BusSelector  OutputSignals=StopsWithinSegment,LongitudinalStoppingDistance,LateralStoppingDistance
  Bus Selector3 : BusSelector  OutputSignals=P0Longitudinal,P1Longitudinal,P2Longitudinal
  Bus Selector4 : BusSelector  OutputSignals=P0Lateral,P1Lateral,P2Lateral
  InitialControlPointsCalculation : Subsystem
  MATLAB Function : MATLAB Function  TreatAsAtomicUnit=on
  ControlPoints : Outport
  wires:
  VehicleMotionT0/1 -> [VehicleMotionT0], Bus Selector/1
  Bus Selector/1 -> InitialControlPointsCalculation/1(PositionLongitudinal)  "<PositionLongitudinal>"
  Bus Selector/2 -> InitialControlPointsCalculation/2(VelocityLongitudinal)  "<VelocityLongitudinal>"
  Bus Selector/3 -> InitialControlPointsCalculation/3(AccelerationLongitudinal)  "<AccelerationLongitudinal>"
  Bus Selector/4 -> InitialControlPointsCalculation/4(PositionLateral)  "<PositionLateral>"
  Bus Selector/5 -> InitialControlPointsCalculation/5(VelocityLateral)  "<VelocityLateral>"
  Bus Selector/6 -> InitialControlPointsCalculation/6(AccelerationLateral)  "<AccelerationLateral>"
  StoppingDistances/1 -> Bus Selector2/1, InitialControlPointsCalculation/7(StoppingDistances)
  [VehicleMotionT0] -> Bus Selector1/1
  Bus Selector1/1 -> MATLAB Function/1(PositionLongitudinal)  "<PositionLongitudinal>"
  Bus Selector1/2 -> MATLAB Function/2(PositionLateral)  "<PositionLateral>"
  Bus Selector1/3 -> MATLAB Function/3(V)  "<Speed>"
  Bus Selector1/4 -> MATLAB Function/4(A)  "<A>"
  Bus Selector1/5 -> MATLAB Function/5(HeadingAngle)  "<HeadingAngle>"
  Bus Selector1/6 -> MATLAB Function/6(Curvature)  "<Curvature>"
  Bus Selector1/7 -> MATLAB Function/7(CurvatureRate)  "<CurvatureRate>"
  Bus Selector2/1 -> MATLAB Function/8(StopsWithinSegment)  "<StopsWithinSegment>"
  Bus Selector2/2 -> MATLAB Function/9(LongitudinalStoppingDistance)  "<LongitudinalStoppingDistance>"
  Bus Selector2/3 -> MATLAB Function/10(LateralStoppingDistance)  "<LateralStoppingDistance>"
  InitialControlPointsCalculation/1(InitialControlPoints) -> [InitialControlPoints], Bus Selector3/1
  MATLAB Function/1(P3Longitudinal) -> Bus Creator/4
  MATLAB Function/2(P3Lateral) -> Bus Creator/8
  Bus Selector3/1 -> Bus Creator/1  "<P0Longitudinal>"
  Bus Selector3/2 -> Bus Creator/2  "<P1Longitudinal>"
  Bus Selector3/3 -> Bus Creator/3  "<P2Longitudinal>"
  [InitialControlPoints] -> Bus Selector4/1
  Bus Selector4/2 -> Bus Creator/6  "<P1Lateral>"
  Bus Selector4/1 -> Bus Creator/5  "<P0Lateral>"
  Bus Selector4/3 -> Bus Creator/7  "<P2Lateral>"
  Bus Creator/1 -> ControlPoints/1  "ControlPoints"

== system TosAeb/AEB_Objsel/TA_Processor/PreProcessor/VehiclePreProcessor/PathEstimn_Calculate/For Iterator PahEstimn/PahEstimn1 ==
  ControlPoints : Inport
  Index : Inport  Port=2
  Assignment : Assignment  OutputInitialize=Specify size for each dimension in table  IndexOptions=Index vector (port)  Indices=1  OutputSizes=4
  Bus Creator : BusCreator  Inputs=3  OutDataTypeStr=Bus: PahEstimn  NonVirtualBus=on
  Bus Selector : BusSelector  OutputSignals=P0Lateral,P1Lateral,P2Lateral,P3Lateral,P0Longitudinal,P1Longitudinal,P2Longitudinal,P3Longitudinal
  Compare To Constant : simulink/Logic and Bit Operations/Compare To Constant  relop===  const=1  OutDataTypeStr=boolean
  Constant : Constant  Value=[1,2,3,4]  VectorParams1D=off
  Data Type Conversion : DataTypeConversion
  Selector : Selector  InputPortWidth=4  IndexOptions=Index vector (port)  Indices=[1 3]  OutputSizes=1
  Switch : Switch  Criteria=u2 ~= 0
  Unit Delay : UnitDelay  SampleTime=-1  HasFrameUpgradeWarning=on
  Vector Concatenate : Concatenate  NumInputs=4
  Vector Concatenate2 : Concatenate
  Vector Concatenate1 : Concatenate  NumInputs=4
  Zero : Constant  Value=0  OutDataTypeStr=Inherit: Inherit via back propagation
  PahEstimn : Outport  PortDimensions=4
  wires:
  ControlPoints/1 -> Bus Selector/1
  Bus Selector/1 -> Vector Concatenate/1  "<P0Lateral>"
  Bus Selector/2 -> Vector Concatenate/2  "<P1Lateral>"
  Bus Selector/3 -> Vector Concatenate/3  "<P2Lateral>"
  Bus Selector/4 -> Vector Concatenate/4  "<P3Lateral>"
  Bus Selector/5 -> Vector Concatenate1/1  "<P0Longitudinal>"
  Bus Selector/6 -> Vector Concatenate1/2  "<P1Longitudinal>"
  Bus Selector/7 -> Vector Concatenate1/3  "<P2Longitudinal>"
  Bus Selector/8 -> Vector Concatenate1/4  "<P3Longitudinal>"
  Index/1 -> Assignment/2, [Index], Compare To Constant/1, Selector/2
  Constant/1 -> Selector/1
  Compare To Constant/1 -> Switch/2  "FirstSegment"
  Zero/1 -> Switch/1
  Selector/1 -> Unit Delay/1, Vector Concatenate2/2  "T1"
  Unit Delay/1 -> Switch/3
  Switch/1 -> Vector Concatenate2/1  "T0"
  Vector Concatenate/1 -> Bus Creator/1  "CtrlAryLat"
  Vector Concatenate1/1 -> Bus Creator/2  "CtrlAryLgt"
  Vector Concatenate2/1 -> Data Type Conversion/1
  Bus Creator/1 -> Assignment/1  "PahEstimn"
  Assignment/1 -> PahEstimn/1  "PahEstimn"
  Data Type Conversion/1 -> Bus Creator/3  "TiAry"
